simulink model "GLOSA_cs"  (Simulink 7.6)
settings: Solver=ode5  SolverMode=SingleTasking  StartTime=0.0  StopTime=40  FixedStep=1/100  RelTol=1e-3
PreLoadFcn:
  | check_NUTS_defined;
  | 
  | assignin('base',['model_closed_' bdroot],false);
InitFcn:
  | PreScanExperimentInitFcn(bdroot)
StopFcn:
  | PreScanExperimentStopFcn(bdroot)
undefined symbols (not defined in the file or in its folder): acceleration, actorID, actorType, DiscIntNeverNeededParam, GPS_alt, GPS_lat, GPS_long, intmax, lightBarInUse, messageID, publicVehicleType, rotZ, sireneInUse, trafficLightPriority, turnAdvice, velocity, x_ini_VRML, y_ini_VRML, yawRate, z_ini_VRML
libraries: simulink x337, simulink_extras x42, PreScanSimulinkLibrary x38, PreScanUsefulBlocks x14, simulink_need_slupdate x6
(part 3 of 38)

== system GLOSA_cs/Ford_Fiesta_1 ==
  SELF_Ford_Fiesta_1 : Inport  Tag=SELFInport
  Antenna_Rx_10 : Inport  Tag=AntennaRInport  Port=2
  Antenna_Rx_10_BusSelector : BusSelector  Tag=BusAntennaR  OutputSignals=warning,messageID,actorID,actorType,publicVehicleType,lightBarInUse,sireneInUse,velocity,acceleration,yawRate,turnAdvice,trafficLightPriority,GPS_lat,GPS_long,GPS_alt,rotZ
  Antenna_Rx_10_Demux : Subsystem  Tag=DemuxAntennaR  mask(PreScan Demux Block:){Channel=1}
      settings: DeleteFcn=PreScanBlockDeleteFcn;
      mask Display:
      | fprintf('Channel: %d',Channel)
      | port_label('output', 1, 'Vehicle_ID')
      | port_label('output', 2, 'GPS Pos Lat [deg]')
      | port_label('output', 3, 'GPS Pos Long [deg]')
      | port_label('output', 4, 'GPS Pos Alt [m]')
      | port_label('output', 5, 'Velocity [m/s]')
      | port_label('output', 6, 'Acceleration [m/s2]')
      | port_label('output', 7, 'Heading [deg]')
      | port_label('output', 8, 'Warning signal ID')
      | port_label('output', 9, 'Maneuvre ID')
      | port_label('output', 10, 'Vehicle Type')
      | port_label('output', 11, 'Braking Capacity')
      | port_label('output', 12, 'Vehicle Size')
      | port_label('output', 13, 'Spare')
  Antenna_Tx_10_BusAssignment : BusAssignment  Tag=BusAntennaT  AssignedSignals=warning,messageID,actorID,actorType,publicVehicleType,lightBarInUse,sireneInUse,velocity,acceleration,yawRate,turnAdvice,trafficLightPriority,GPS_lat,GPS_long,GPS_alt,rotZ
  Antenna_Tx_10_Mux : Subsystem  Tag=MuxAntennaT  mask(PreScan Mux Block:){Channel=1, TimeStep=1/100}
      mask prompts: TimeStep "Time Step [s]"
      settings: DeleteFcn=PreScanBlockDeleteFcn;
      mask Display:
      | fprintf('Channel: %d',Channel)
      | port_label('input', 1, 'Warning signal ID')
      | port_label('input', 2, 'Maneuvre ID')
      | port_label('input', 3, 'Vehicle Type')
      | port_label('input', 4, 'Braking Capacity')
      | port_label('input', 5, 'Vehicle Size')
      | port_label('input', 6, 'spare')
  Bus Selector : BusSelector  OutputSignals=Brake_[bar]
  Compare To Constant : simulink/Logic and Bit Operations/Compare To Constant  SystemSampleTime=-1  relop=>=  const=0.1  LogicOutDataTypeMode=uint8
  Constant1 : Constant  Value=15
  Constant2 : Constant  Value=15
  Data Type Conversion : DataTypeConversion
  Dynamics_Simple : Subsystem  Tag=Simple_Vehicle_Dynamics  mask(PreScan Block: Simple Dynamics){Migrate=off, TimeStep=1/100, ComputationMode=Continuous_Time}
      mask prompts: Migrate "Migrate. During migration this block will be replaced by a newer one."; TimeStep "Auto assigned: Time Step [s]"; ComputationMode "Computation mode:"
      mask help:
      | The Simple Dynamics Model is selected in the GUI to simulate vehicle dynamics behavior.
      |
      | The Simple Dynamics Model is completely open to the user. The parameters of this model can be set in the Simple Dynamics dialog in the GUI.
      |
      | For more information, please click Help.
      mask init:
      | p = SimpleDyn_pars;
      settings: DeleteFcn=PreScanBlockDeleteFcn;
      mask Help: eval('openHelp(220)')
      mask Display:
      | image(imread('SimpleDyn.png'))
      | port_label('input', 1, '{\delta}_S_t_ _e_e_r [deg]','texmode','on')
      | port_label('input', 2, 'Throttle [%]')
      | port_label('input', 3, 'Brake Pres [bar]')
      | port_label('input', 4, 'V_0 [ms-1]','texmode','on')
      | port_label('input', 5, 'Auto=[0]/Manual=[1]')
      | port_label('input', 6, 'Auto_GearShift')
      | port_label('input', 7, 'Manual_GearShift')
      | port_label('output', 1, 'State')
      | port_label('output', 2, 'Vx [ms-1]')
      | port_label('output', 3, 'Perf Params')
  GLOSA : Subsystem
  Gain : Gain  Gain=1/20
  Gain1 : Gain  Gain=1/20
  Ground_{1..22} (22 alike) : Ground
  Integer Delay{1..5} (5 alike) : simulink/Discrete/Integer Delay  NumDelays=1  InputProcessing=Inherited  vinit=0.0  samptime=-1
  Mux : Mux  Inputs=3
  PathFollower_1 : Subsystem  Tag=pathFollower  mask(PreScan Block: Path Follower){Migrate=on, FileName='Trajectory_4.mat', TunableSteeringGain=1, XShifting_wrt_COG=0}
      mask prompts: Migrate "Migrate. During migration this block will be replaced by a newer one."; FileName "Trajectory Name"; TunableSteeringGain "TunableSteeringGain [-]"; XShifting_wrt_COG "XShifting_wrt_COG [m]"
      mask help:
      | The Path Follower Model is selected in the GUI to simulate driver behavior.
      |
      | For more information, please click Help.
      mask init:
      | load(['trajectories/',eval(FileName)])
      | traject=trajectory';
      | 
      | Traj_x=traject(:,2);
      | Traj_y=traject(:,3);
      | Traj_z=traject(:,4);
      | Traj_vx=traject(:,8);
      | Traj_vy=traject(:,9);
      | Traj_vz=traject(:,10);
      mask Help: eval('openHelp(232)')
  Rate Limiter1 : RateLimiter  RisingSlewLimit=1.5  FallingSlewLimit=-1.5  SampleTimeMode=inherited  InitialCondition=15
  SELF_Demux : Subsystem  Tag=DemuxSELF
      settings: DeleteFcn=PreScanBlockDeleteFcn;
      mask Type: PreScan Demux Block:
      mask Display:
      | port_label('output',1,'x [m]')
      | port_label('output',2,'y [m]')
      | port_label('output',3,'z [m]','texmode','on')
      | port_label('output',4,'Rotx [deg]','texmode','on')
      | port_label('output',5,'Roty [deg]','texmode','on')
      | port_label('output',6,'Rotz [deg]','texmode','on')
      | port_label('output',7,'GPS lat [deg]','texmode','on')
      | port_label('output',8,'GPS long [deg]','texmode','on')
      | port_label('output',9,'GPS alt [m]','texmode','on')
      | port_label('output',10,'Velocity [m/s]')
      | port_label('output',11,'Heading [deg]')
      | port_label('output',12,'Yaw rate [deg/s]')
  Subsystem : Subsystem
  Terminator_{1..19} (19 alike) : Terminator
  TextureColor : Constant  Tag=TextureColor  Value="[0  0  0]"
  Trajectories : Subsystem  Tag=Trajectories
      settings: DeleteFcn=PreScanBlockDeleteFcn;
      mask Type: PreScan Source Block: Trajectories
      mask Description:
      | The Trajectory block contains the trajectories defined in the PreScan Experiment Editor. The output of the trajectory block is the (vehicle) State.
      | 
      | For more information, please click Help.
      mask Help: eval('openHelp(312)')
      mask Display: image(imread('Trajectory.png'));
  VISULightSource_Mux : Subsystem  Tag=MuxLightSource
      settings: DeleteFcn=PreScanBlockDeleteFcn;
      mask Type: PreScan Mux Block:
  VISUSteer_Mux : Subsystem  Tag=MuxSteer
      settings: DeleteFcn=PreScanBlockDeleteFcn;
      mask Type: PreScan Mux Block:
  VISUTextureColor_Mux : Subsystem  Tag=MuxSteer
      settings: DeleteFcn=PreScanBlockDeleteFcn;
      mask Type: PreScan Mux Block:
  VISUWheels_Mux : Subsystem  Tag=MuxWheels  mask(PreScan Mux Block:){TimeStep=1/100}
      mask prompts: TimeStep "Auto Assigned: Time Step [s]"
      settings: DeleteFcn=PreScanBlockDeleteFcn;
  pos : Constant  Tag=pos  Value=[271.5 5.25 180]
  vehicle CAM generator : Subsystem
  wheel Z disp : Subsystem  mask(Simple Dynamics parameters){a=1.17, b=1.77}
      mask prompts: a "Distance to the front wheel from cars center of gravity a [m]"; b "Distance to the back wheel from cars center of gravity b [m]"
  STATE_Ford_Fiesta_1 : Outport  Tag=StateOutport
  Antenna_Tx_10 : Outport  Tag=AntennaTOutport  Port=2
  VISULightSource_Ford_Fiesta_1 : Outport  Tag=VisuLightsOutport  Port=3  SampleTime=1/100
  VISUTextureColor_Ford_Fiesta_1 : Outport  Tag=VisuTextureColorOutport  Port=4  SampleTime=1/100
  VISUWheels_Ford_Fiesta_1 : Outport  Tag=VisuWheelsOutport  Port=5  SampleTime=1/100
  VISUSteer_Ford_Fiesta_1 : Outport  Tag=VisuSteerOutport  Port=6  SampleTime=1/100
  wires:
  Constant2/1 -> GLOSA/23(desiredVelocity)
  [Ford_Fiesta_1_GPS] -> vehicle CAM generator/10(GPS_Noise_src)
  [Ford_Fiesta_1_MUR] -> vehicle CAM generator/11(MessageUpdateRate)
  Bus Selector/1 -> Compare To Constant/1  "<Brake_[bar]>"
  Compare To Constant/1 -> Data Type Conversion/1
  [Ford_Fiesta_1_NOMM] -> GLOSA/24(NoMissedMessages)
  [Ford_Fiesta_1_TD] -> GLOSA/25(MessageDelay)
  GLOSA/1(velocity) -> Rate Limiter1/1
  Gain/1 -> Integer Delay3/1
  [Steer] -> Gain/1
  Gain1/1 -> Integer Delay2/1
  [Steer] -> Gain1/1
  [Vx_ms] -> Integer Delay5/1
  [Roty] -> wheel Z disp/1(rotY [deg])
  Rate Limiter1/1 -> Integer Delay4/1
  [Params] -> Bus Selector/1
  pos/1 -> PathFollower_1/1(TrajOffset_x,y,Y [m,m,deg])
  SELF_Ford_Fiesta_1/1 -> SELF_Demux/1(SELF)
  Antenna_Rx_10_Demux/13(spare) -> Antenna_Rx_10_BusSelector/1
  Antenna_Rx_10/1 -> Antenna_Rx_10_Demux/1(AntennaR)
  Antenna_Tx_10_Mux/2(Out2) -> [Antenna_Tx_10]
  [Antenna_Tx_10] -> Antenna_Tx_10_BusAssignment/1
  Antenna_Tx_10_Mux/1(Out1) -> Antenna_Tx_10/1
  VISULightSource_Mux/1(Lights) -> VISULightSource_Ford_Fiesta_1/1
  VISUTextureColor_Mux/1(TextureColor) -> VISUTextureColor_Ford_Fiesta_1/1
  TextureColor/1 -> VISUTextureColor_Mux/1(RGB [-,-,-])
  VISUWheels_Mux/1(Wheels) -> VISUWheels_Ford_Fiesta_1/1
  VISUSteer_Mux/1(Steer) -> VISUSteer_Ford_Fiesta_1/1
  PathFollower_1/2(throttle [%]) -> Dynamics_Simple/2(Throttle)
  PathFollower_1/3(brake pressure [bar]) -> Dynamics_Simple/3(Brake)
  PathFollower_1/4(v0 [m/s]) -> Dynamics_Simple/4(Initial_Vx_[ms-1])
  PathFollower_1/5(Auto_GearShift) -> Dynamics_Simple/6(Auto_GearShift)
  PathFollower_1/1(delta_steerwheel [deg]) -> Dynamics_Simple/1(DeltaSteer_[deg]), [Steer]
  vehicle CAM generator/16(CAM_rotZ) -> Antenna_Tx_10_BusAssignment/17
  vehicle CAM generator/15(CAM_GPSalt) -> Antenna_Tx_10_BusAssignment/16
  vehicle CAM generator/14(CAM_GPSlong) -> Antenna_Tx_10_BusAssignment/15
  vehicle CAM generator/13(CAM_GPSlat) -> Antenna_Tx_10_BusAssignment/14
  vehicle CAM generator/12(CAM_trafficLightPriority) -> Antenna_Tx_10_BusAssignment/13
  vehicle CAM generator/11(CAM_turnAdvice) -> Antenna_Tx_10_BusAssignment/12
  vehicle CAM generator/10(CAM_yawRate) -> Antenna_Tx_10_BusAssignment/11
  Antenna_Rx_10_BusSelector/16 -> GLOSA/22(CAM_rotZ_in)  "<rotZ>"
  Antenna_Rx_10_BusSelector/15 -> GLOSA/21(CAM_GPSalt_in)  "<GPS_alt>"
  Antenna_Rx_10_BusSelector/14 -> GLOSA/20(CAM_GPSlong_in)  "<GPS_long>"
  Antenna_Rx_10_BusSelector/13 -> GLOSA/19(CAM_GPSlat_in)  "<GPS_lat>"
  Antenna_Rx_10_BusSelector/12 -> GLOSA/18(CAM_trafficLightPriority_in)  "<trafficLightPriority>"
  Antenna_Rx_10_BusSelector/11 -> GLOSA/17(CAM_turnAdvice_in)  "<turnAdvice>"
  Antenna_Rx_10_BusSelector/10 -> GLOSA/16(CAM_yawRate_in)  "<yawRate>"
  Antenna_Rx_10_BusSelector/9 -> GLOSA/15(CAM_acceleration_in)  "<acceleration>"
  Antenna_Rx_10_BusSelector/8 -> GLOSA/14(CAM_velocity_in)  "<velocity>"
  Antenna_Rx_10_BusSelector/7 -> GLOSA/13(CAM_sireneInUse_in)  "<sireneInUse>"
  Antenna_Rx_10_BusSelector/6 -> GLOSA/12(CAM_lightBarInUse_in)  "<lightBarInUse>"
  Antenna_Rx_10_BusSelector/5 -> GLOSA/11(CAM_publicVehicleType_in)  "<publicVehicleType>"
  Antenna_Rx_10_BusSelector/4 -> GLOSA/10(CAM_vehicleType_in)  "<actorType>"
  Antenna_Rx_10_BusSelector/3 -> GLOSA/9(CAM_vehicleID_in)  "<actorID>"
  Antenna_Rx_10_BusSelector/2 -> GLOSA/8(CAM_messageID_in)  "<messageID>"
  Antenna_Rx_10_BusSelector/1 -> GLOSA/7(CAM_warning_in)  "<warning>"
  Constant1/1 -> PathFollower_1/4(Initial Velocity [m/s])
  Subsystem/1(Out1) -> STATE_Ford_Fiesta_1/1
  Mux/1 -> PathFollower_1/3(position x,y,Y [m,m,deg])
  SELF_Demux/12(yaw rate [deg/s]) -> vehicle CAM generator/9(yawRate), GLOSA/6(selfYawRate)
  SELF_Demux/10(velocity [m/s]) -> PathFollower_1/6(velocity [m/s]), vehicle CAM generator/8(velocity [m/s]), GLOSA/5(selfVelocity)
  SELF_Demux/9(GPS alt [m]) -> vehicle CAM generator/7(GPS_alt), GLOSA/3(selfGPSAltitude)
  SELF_Demux/8(GPS long [deg]) -> vehicle CAM generator/6(GPS_long), GLOSA/2(selfGPSLongitude)
  SELF_Demux/7(GPS lat [deg]) -> vehicle CAM generator/5(GPS_lat), GLOSA/1(selfGPSLatitude)
  SELF_Demux/6(rotz [deg]) -> Mux/3, vehicle CAM generator/4(rotZ [deg]), GLOSA/4(selfRotZ)
  SELF_Demux/3(z [m]) -> vehicle CAM generator/3(z [m])
  SELF_Demux/2(y [m]) -> Mux/2, vehicle CAM generator/2(y [m])
  SELF_Demux/1(x [m]) -> Mux/1, vehicle CAM generator/1(x [m])
  vehicle CAM generator/1(CAM_warning) -> Antenna_Tx_10_BusAssignment/2
  vehicle CAM generator/2(CAM_messageID) -> Antenna_Tx_10_BusAssignment/3
  vehicle CAM generator/3(CAM_vehicleID) -> Antenna_Tx_10_BusAssignment/4
  vehicle CAM generator/4(CAM_vehicleType) -> Antenna_Tx_10_BusAssignment/5
  vehicle CAM generator/5(CAM_publicVehicleType) -> Antenna_Tx_10_BusAssignment/6
  vehicle CAM generator/6(CAM_lightBarInUse) -> Antenna_Tx_10_BusAssignment/7
  vehicle CAM generator/7(CAM_sireneInUse) -> Antenna_Tx_10_BusAssignment/8
  vehicle CAM generator/8(CAM_velocity) -> Antenna_Tx_10_BusAssignment/9
  vehicle CAM generator/9(CAM_acceleration) -> Antenna_Tx_10_BusAssignment/10
  wheel Z disp/1(zDisp FL [m]) -> VISUWheels_Mux/4(zDisp FL [m])
  wheel Z disp/2(zDisp FR [m]) -> VISUWheels_Mux/5(zDisp FR [m])
  wheel Z disp/3(zDisp RL [m]) -> VISUWheels_Mux/6(zDisp RL [m])
  wheel Z disp/4(zDisp RR [m]) -> VISUWheels_Mux/7(zDisp RR [m])
  Data Type Conversion/1 -> VISULightSource_Mux/15(CombinedBrakeLightRight), VISULightSource_Mux/14(CombinedBrakeLightLeft), VISULightSource_Mux/11(BrakeLightMiddle)
  Integer Delay2/1 -> VISUWheels_Mux/1(Steer Angle Front [deg])
  Integer Delay5/1 -> VISUWheels_Mux/3(Velocity [m/s])
  Integer Delay3/1 -> VISUSteer_Mux/2(Steer [deg])
  SELF_Demux/5(roty [deg]) -> [Roty]
  Dynamics_Simple/3(Perf_Params) -> [Params]
  Integer Delay1/1 -> Antenna_Tx_10_Mux/6(spare)
  Integer Delay4/1 -> PathFollower_1/5(Desired Velocity [m/s])
  Dynamics_Simple/1(State) -> Subsystem/1(In1)
  Dynamics_Simple/2(Vx [m s-1]) -> [Vx_ms]
  Antenna_Tx_10_BusAssignment/1 -> Integer Delay1/1
  Antenna_Rx_10_Demux/1(Vehicle_ID) -> Terminator_1/1
  Antenna_Rx_10_Demux/2(GPS_pos_lat_[deg]) -> Terminator_2/1
  Antenna_Rx_10_Demux/3(GPS_pos_long_[deg]) -> Terminator_3/1
  Antenna_Rx_10_Demux/4(GPS_pos_alt_[m]) -> Terminator_4/1
  Antenna_Rx_10_Demux/5(Velocity_[m/s]) -> Terminator_5/1
  Antenna_Rx_10_Demux/6(Acceleration_[m/s2]) -> Terminator_6/1
  Antenna_Rx_10_Demux/7(Heading [deg]) -> Terminator_7/1
  Antenna_Rx_10_Demux/8(warning_signal_id) -> Terminator_8/1
  Antenna_Rx_10_Demux/9(maneuvre_id) -> Terminator_9/1
  Antenna_Rx_10_Demux/10(vehicle_type) -> Terminator_10/1
  Antenna_Rx_10_Demux/11(brakingcapacity) -> Terminator_11/1
  Antenna_Rx_10_Demux/12(vehicle_size) -> Terminator_12/1
  Ground_1/1 -> Antenna_Tx_10_Mux/1(warning_signal_id)
  Ground_2/1 -> Antenna_Tx_10_Mux/2(maneuvre_id)
  Ground_3/1 -> Antenna_Tx_10_Mux/3(vehicle_type)
  Ground_4/1 -> Antenna_Tx_10_Mux/4(brakingcapacity)
  Ground_5/1 -> Antenna_Tx_10_Mux/5(vehicle_size)
  Ground_6/1 -> Dynamics_Simple/5(AutoManual_OnOff)
  Ground_7/1 -> Dynamics_Simple/7(Manual_GearShift)
  GLOSA/2(Time) -> Terminator_13/1
  GLOSA/3(Light) -> Terminator_14/1
  Ground_8/1 -> PathFollower_1/2(ActorOffset_Left(+) / Right(-) [m])
  PathFollower_1/6(Actor/Traj_distance [m]) -> Terminator_15/1
  PathFollower_1/7(Actor/Traj_angle [deg]) -> Terminator_16/1
  SELF_Demux/4(rotx [deg]) -> Terminator_17/1
  SELF_Demux/11(heading [deg]) -> Terminator_18/1
  Trajectories/1(State) -> Terminator_19/1
  Ground_9/1 -> VISULightSource_Mux/1(IndicatorLightsLeft)
  Ground_10/1 -> VISULightSource_Mux/2(IndicatorLightsRight)
  Ground_11/1 -> VISULightSource_Mux/3(FogLightRearLeft)
  Ground_12/1 -> VISULightSource_Mux/4(FogLightRearRight)
  Ground_13/1 -> VISULightSource_Mux/5(FogLightFrontLeft)
  Ground_14/1 -> VISULightSource_Mux/6(FogLightFrontRight)
  Ground_15/1 -> VISULightSource_Mux/7(MainLightRearLeft)
  Ground_16/1 -> VISULightSource_Mux/8(MainLightRearRight)
  Ground_17/1 -> VISULightSource_Mux/9(MainLightFrontLeft)
  Ground_18/1 -> VISULightSource_Mux/10(MainLightFrontRight)
  Ground_19/1 -> VISULightSource_Mux/12(ReverseLightLeft)
  Ground_20/1 -> VISULightSource_Mux/13(ReverseLightRight)
  Ground_21/1 -> VISUSteer_Mux/1(Column [deg])
  Ground_22/1 -> VISUWheels_Mux/2(Steer Angle Rear [deg])

== system GLOSA_cs/GetSensors ==
  dummy : Inport
  GetSensors : M-S-Function  FunctionName=GetSensorsBlock  Parameters=TimeStep NrOfSensors
  tag scope: SimCoreSensors=global
  wires:
  GetSensors/1 -> [SimCoreSensors]
  dummy/1 -> GetSensors/1

== system GLOSA_cs/Mazda_RX8_1 ==
  SELF_Mazda_RX8_1 : Inport  Tag=SELFInport
  Antenna_Rx_8 : Inport  Tag=AntennaRInport  Port=2
  Antenna_Rx_8_BusSelector : BusSelector  Tag=BusAntennaR  OutputSignals=warning,messageID,actorID,actorType,publicVehicleType,lightBarInUse,sireneInUse,velocity,acceleration,yawRate,turnAdvice,trafficLightPriority,GPS_lat,GPS_long,GPS_alt,rotZ
  Antenna_Rx_8_Demux : Subsystem  Tag=DemuxAntennaR  mask(PreScan Demux Block:){Channel=1}
      settings: DeleteFcn=PreScanBlockDeleteFcn;
      mask Display:
      | fprintf('Channel: %d',Channel)
      | port_label('output', 1, 'Vehicle_ID')
      | port_label('output', 2, 'GPS Pos Lat [deg]')
      | port_label('output', 3, 'GPS Pos Long [deg]')
      | port_label('output', 4, 'GPS Pos Alt [m]')
      | port_label('output', 5, 'Velocity [m/s]')
      | port_label('output', 6, 'Acceleration [m/s2]')
      | port_label('output', 7, 'Heading [deg]')
      | port_label('output', 8, 'Warning signal ID')
      | port_label('output', 9, 'Maneuvre ID')
      | port_label('output', 10, 'Vehicle Type')
      | port_label('output', 11, 'Braking Capacity')
      | port_label('output', 12, 'Vehicle Size')
      | port_label('output', 13, 'Spare')
  Antenna_Tx_8_BusAssignment : BusAssignment  Tag=BusAntennaT  AssignedSignals=warning,messageID,actorID,actorType,publicVehicleType,lightBarInUse,sireneInUse,velocity,acceleration,yawRate,turnAdvice,trafficLightPriority,GPS_lat,GPS_long,GPS_alt,rotZ
  Antenna_Tx_8_Mux : Subsystem  Tag=MuxAntennaT  mask(PreScan Mux Block:){Channel=1, TimeStep=1/100}
      mask prompts: TimeStep "Time Step [s]"
      settings: DeleteFcn=PreScanBlockDeleteFcn;
      mask Display:
      | fprintf('Channel: %d',Channel)
      | port_label('input', 1, 'Warning signal ID')
      | port_label('input', 2, 'Maneuvre ID')
      | port_label('input', 3, 'Vehicle Type')
      | port_label('input', 4, 'Braking Capacity')
      | port_label('input', 5, 'Vehicle Size')
      | port_label('input', 6, 'spare')
  Bus Selector : BusSelector  OutputSignals=Brake_[bar]
  Compare To Constant : simulink/Logic and Bit Operations/Compare To Constant  SystemSampleTime=-1  relop=>=  const=0.1  LogicOutDataTypeMode=uint8
  Constant1 : Constant  Value=15
  Constant2 : Constant  Value=15
  Data Type Conversion : DataTypeConversion
  Dynamics_Simple : Subsystem  Tag=Simple_Vehicle_Dynamics  mask(PreScan Block: Simple Dynamics){Migrate=off, TimeStep=1/100, ComputationMode=Continuous_Time}
      mask prompts: Migrate "Migrate. During migration this block will be replaced by a newer one."; TimeStep "Auto assigned: Time Step [s]"; ComputationMode "Computation mode:"
      mask help:
      | The Simple Dynamics Model is selected in the GUI to simulate vehicle dynamics behavior.
      |
      | The Simple Dynamics Model is completely open to the user. The parameters of this model can be set in the Simple Dynamics dialog in the GUI.
      |
      | For more information, please click Help.
      mask init:
      | p = SimpleDyn_pars;
      settings: DeleteFcn=PreScanBlockDeleteFcn;
      mask Help: eval('openHelp(220)')
      mask Display:
      | image(imread('SimpleDyn.png'))
      | port_label('input', 1, '{\delta}_S_t_ _e_e_r [deg]','texmode','on')
      | port_label('input', 2, 'Throttle [%]')
      | port_label('input', 3, 'Brake Pres [bar]')
      | port_label('input', 4, 'V_0 [ms-1]','texmode','on')
      | port_label('input', 5, 'Auto=[0]/Manual=[1]')
      | port_label('input', 6, 'Auto_GearShift')
      | port_label('input', 7, 'Manual_GearShift')
      | port_label('output', 1, 'State')
      | port_label('output', 2, 'Vx [ms-1]')
      | port_label('output', 3, 'Perf Params')
  GLOSA : Subsystem
  Gain : Gain  Gain=1/20
  Gain1 : Gain  Gain=1/20
  Ground_{1..20} (20 alike) : Ground
  Integer Delay{1..5} (5 alike) : simulink/Discrete/Integer Delay  NumDelays=1  InputProcessing=Inherited  vinit=0.0  samptime=-1
  Mux : Mux  Inputs=3
  PathFollower_1 : Subsystem  Tag=pathFollower  mask(PreScan Block: Path Follower){Migrate=on, FileName='Trajectory_5.mat', TunableSteeringGain=1, XShifting_wrt_COG=0}
      mask prompts: Migrate "Migrate. During migration this block will be replaced by a newer one."; FileName "Trajectory Name"; TunableSteeringGain "TunableSteeringGain [-]"; XShifting_wrt_COG "XShifting_wrt_COG [m]"
      mask help:
      | The Path Follower Model is selected in the GUI to simulate driver behavior.
      |
      | For more information, please click Help.
      mask init:
      | load(['trajectories/',eval(FileName)])
      | traject=trajectory';
      | 
      | Traj_x=traject(:,2);
      | Traj_y=traject(:,3);
      | Traj_z=traject(:,4);
      | Traj_vx=traject(:,8);
      | Traj_vy=traject(:,9);
      | Traj_vz=traject(:,10);
      mask Help: eval('openHelp(232)')
  Rate Limiter1 : RateLimiter  RisingSlewLimit=1.5  FallingSlewLimit=-1.5  SampleTimeMode=inherited  InitialCondition=15
  Rate Transition2 : RateTransition  Integrity=off
  SELF_Demux : Subsystem  Tag=DemuxSELF
      settings: DeleteFcn=PreScanBlockDeleteFcn;
      mask Type: PreScan Demux Block:
      mask Display:
      | port_label('output',1,'x [m]')
      | port_label('output',2,'y [m]')
      | port_label('output',3,'z [m]','texmode','on')
      | port_label('output',4,'Rotx [deg]','texmode','on')
      | port_label('output',5,'Roty [deg]','texmode','on')
      | port_label('output',6,'Rotz [deg]','texmode','on')
      | port_label('output',7,'GPS lat [deg]','texmode','on')
      | port_label('output',8,'GPS long [deg]','texmode','on')
      | port_label('output',9,'GPS alt [m]','texmode','on')
      | port_label('output',10,'Velocity [m/s]')
      | port_label('output',11,'Heading [deg]')
      | port_label('output',12,'Yaw rate [deg/s]')
  Subsystem : Subsystem
  Terminator_{1..17} (17 alike) : Terminator
  TextureColor : Constant  Tag=TextureColor  Value="[255    0    0]"
  Trajectories : Subsystem  Tag=Trajectories
      settings: DeleteFcn=PreScanBlockDeleteFcn;
      mask Type: PreScan Source Block: Trajectories
      mask Description:
      | The Trajectory block contains the trajectories defined in the PreScan Experiment Editor. The output of the trajectory block is the (vehicle) State.
      | 
      | For more information, please click Help.
      mask Help: eval('openHelp(312)')
      mask Display: image(imread('Trajectory.png'));
  V2x_TC_GUI : Subsystem
  VISULightSource_Mux : Subsystem  Tag=MuxLightSource
      settings: DeleteFcn=PreScanBlockDeleteFcn;
      mask Type: PreScan Mux Block:
  VISUSteer_Mux : Subsystem  Tag=MuxSteer
      settings: DeleteFcn=PreScanBlockDeleteFcn;
      mask Type: PreScan Mux Block:
  VISUTextureColor_Mux : Subsystem  Tag=MuxSteer
      settings: DeleteFcn=PreScanBlockDeleteFcn;
      mask Type: PreScan Mux Block:
  VISUWheels_Mux : Subsystem  Tag=MuxWheels  mask(PreScan Mux Block:){TimeStep=1/100}
      mask prompts: TimeStep "Auto Assigned: Time Step [s]"
      settings: DeleteFcn=PreScanBlockDeleteFcn;
  pos : Constant  Tag=pos  Value=[-271.5 -5.2499999999999671 0]
  vehicle CAM generator : Subsystem
  wheel Z disp : Subsystem  mask(Simple Dynamics parameters){a=1.17, b=1.77}
      mask prompts: a "Distance to the front wheel from cars center of gravity a [m]"; b "Distance to the back wheel from cars center of gravity b [m]"
  STATE_Mazda_RX8_1 : Outport  Tag=StateOutport
  Antenna_Tx_8 : Outport  Tag=AntennaTOutport  Port=2
  VISULightSource_Mazda_RX8_1 : Outport  Tag=VisuLightsOutport  Port=3  SampleTime=1/100
  VISUTextureColor_Mazda_RX8_1 : Outport  Tag=VisuTextureColorOutport  Port=4  SampleTime=1/100
  VISUWheels_Mazda_RX8_1 : Outport  Tag=VisuWheelsOutport  Port=5  SampleTime=1/100
  VISUSteer_Mazda_RX8_1 : Outport  Tag=VisuSteerOutport  Port=6  SampleTime=1/100
  tag scope: perf_RX8=global
  wires:
  Constant2/1 -> GLOSA/23(desiredVelocity)
  [Mazda_RX8_1_GPS] -> vehicle CAM generator/10(GPS_Noise_src)
  [Mazda_RX8_1_MUR] -> vehicle CAM generator/11(MessageUpdateRate)
  [Mazda_RX8_1_NOMM] -> GLOSA/24(NoMissedMessages)
  [Mazda_RX8_1_TD] -> GLOSA/25(MessageDelay)
  Bus Selector/1 -> Compare To Constant/1  "<Brake_[bar]>"
  Compare To Constant/1 -> Data Type Conversion/1
  [perf_RX8] -> Rate Transition2/1
  Rate Transition2/1 -> V2x_TC_GUI/1(perf)
  GLOSA/2(Time) -> V2x_TC_GUI/3(lightsTime)
  GLOSA/3(Light) -> V2x_TC_GUI/2(lightsColor)
  GLOSA/1(velocity) -> Rate Limiter1/1
  Gain/1 -> Integer Delay3/1
  [Steer] -> Gain/1
  Rate Limiter1/1 -> Integer Delay1/1
  Integer Delay1/1 -> V2x_TC_GUI/4(suggestedVelocity), PathFollower_1/5(Desired Velocity [m/s])
  [perf_RX8] -> Bus Selector/1
  Gain1/1 -> Integer Delay2/1
  [Steer] -> Gain1/1
  [Vx_ms] -> Integer Delay5/1
  [Roty] -> wheel Z disp/1(rotY [deg])
  pos/1 -> PathFollower_1/1(TrajOffset_x,y,Y [m,m,deg])
  SELF_Mazda_RX8_1/1 -> SELF_Demux/1(SELF)
  Antenna_Rx_8_Demux/13(spare) -> Antenna_Rx_8_BusSelector/1
  Antenna_Rx_8/1 -> Antenna_Rx_8_Demux/1(AntennaR)
  Antenna_Tx_8_Mux/2(Out2) -> [Antenna_Tx_8]
  [Antenna_Tx_8] -> Antenna_Tx_8_BusAssignment/1
  Antenna_Tx_8_Mux/1(Out1) -> Antenna_Tx_8/1
  VISULightSource_Mux/1(Lights) -> VISULightSource_Mazda_RX8_1/1
  VISUTextureColor_Mux/1(TextureColor) -> VISUTextureColor_Mazda_RX8_1/1
  TextureColor/1 -> VISUTextureColor_Mux/1(RGB [-,-,-])
  VISUWheels_Mux/1(Wheels) -> VISUWheels_Mazda_RX8_1/1
  VISUSteer_Mux/1(Steer) -> VISUSteer_Mazda_RX8_1/1
  PathFollower_1/2(throttle [%]) -> Dynamics_Simple/2(Throttle)
  PathFollower_1/3(brake pressure [bar]) -> Dynamics_Simple/3(Brake)
  PathFollower_1/4(v0 [m/s]) -> Dynamics_Simple/4(Initial_Vx_[ms-1])
  PathFollower_1/5(Auto_GearShift) -> Dynamics_Simple/6(Auto_GearShift)
  PathFollower_1/1(delta_steerwheel [deg]) -> Dynamics_Simple/1(DeltaSteer_[deg]), [Steer]
  vehicle CAM generator/16(CAM_rotZ) -> Antenna_Tx_8_BusAssignment/17
  vehicle CAM generator/15(CAM_GPSalt) -> Antenna_Tx_8_BusAssignment/16
  vehicle CAM generator/14(CAM_GPSlong) -> Antenna_Tx_8_BusAssignment/15
  vehicle CAM generator/13(CAM_GPSlat) -> Antenna_Tx_8_BusAssignment/14
  vehicle CAM generator/12(CAM_trafficLightPriority) -> Antenna_Tx_8_BusAssignment/13
  vehicle CAM generator/11(CAM_turnAdvice) -> Antenna_Tx_8_BusAssignment/12
  vehicle CAM generator/9(CAM_acceleration) -> Antenna_Tx_8_BusAssignment/10
  Antenna_Rx_8_BusSelector/16 -> GLOSA/22(CAM_rotZ_in)  "<rotZ>"
  Antenna_Rx_8_BusSelector/15 -> GLOSA/21(CAM_GPSalt_in)  "<GPS_alt>"
  Antenna_Rx_8_BusSelector/14 -> GLOSA/20(CAM_GPSlong_in)  "<GPS_long>"
  Antenna_Rx_8_BusSelector/13 -> GLOSA/19(CAM_GPSlat_in)  "<GPS_lat>"
  Antenna_Rx_8_BusSelector/12 -> GLOSA/18(CAM_trafficLightPriority_in)  "<trafficLightPriority>"
  Antenna_Rx_8_BusSelector/11 -> GLOSA/17(CAM_turnAdvice_in)  "<turnAdvice>"
  Antenna_Rx_8_BusSelector/10 -> GLOSA/16(CAM_yawRate_in)  "<yawRate>"
  Antenna_Rx_8_BusSelector/9 -> GLOSA/15(CAM_acceleration_in)  "<acceleration>"
  Antenna_Rx_8_BusSelector/8 -> GLOSA/14(CAM_velocity_in)  "<velocity>"
  Antenna_Rx_8_BusSelector/7 -> GLOSA/13(CAM_sireneInUse_in)  "<sireneInUse>"
  Antenna_Rx_8_BusSelector/6 -> GLOSA/12(CAM_lightBarInUse_in)  "<lightBarInUse>"
  Antenna_Rx_8_BusSelector/5 -> GLOSA/11(CAM_publicVehicleType_in)  "<publicVehicleType>"
  Antenna_Rx_8_BusSelector/4 -> GLOSA/10(CAM_vehicleType_in)  "<actorType>"
  Antenna_Rx_8_BusSelector/3 -> GLOSA/9(CAM_vehicleID_in)  "<actorID>"
  Antenna_Rx_8_BusSelector/2 -> GLOSA/8(CAM_messageID_in)  "<messageID>"
  Antenna_Rx_8_BusSelector/1 -> GLOSA/7(CAM_warning_in)  "<warning>"
  Constant1/1 -> PathFollower_1/4(Initial Velocity [m/s])
  Subsystem/1(Out1) -> STATE_Mazda_RX8_1/1
  Mux/1 -> PathFollower_1/3(position x,y,Y [m,m,deg])
  SELF_Demux/12(yaw rate [deg/s]) -> vehicle CAM generator/9(yawRate), GLOSA/6(selfYawRate)
  SELF_Demux/10(velocity [m/s]) -> PathFollower_1/6(velocity [m/s]), vehicle CAM generator/8(velocity [m/s]), GLOSA/5(selfVelocity)
  SELF_Demux/9(GPS alt [m]) -> vehicle CAM generator/7(GPS_alt), GLOSA/3(selfGPSAltitude)
  SELF_Demux/8(GPS long [deg]) -> vehicle CAM generator/6(GPS_long), GLOSA/2(selfGPSLongitude)
  SELF_Demux/7(GPS lat [deg]) -> vehicle CAM generator/5(GPS_lat), GLOSA/1(selfGPSLatitude)
  SELF_Demux/6(rotz [deg]) -> Mux/3, vehicle CAM generator/4(rotZ [deg]), GLOSA/4(selfRotZ)
  SELF_Demux/3(z [m]) -> vehicle CAM generator/3(z [m])
  SELF_Demux/2(y [m]) -> Mux/2, vehicle CAM generator/2(y [m])
  SELF_Demux/1(x [m]) -> Mux/1, vehicle CAM generator/1(x [m])
  Dynamics_Simple/3(Perf_Params) -> [perf_RX8]
  vehicle CAM generator/1(CAM_warning) -> Antenna_Tx_8_BusAssignment/2
  vehicle CAM generator/2(CAM_messageID) -> Antenna_Tx_8_BusAssignment/3
  vehicle CAM generator/3(CAM_vehicleID) -> Antenna_Tx_8_BusAssignment/4
  vehicle CAM generator/4(CAM_vehicleType) -> Antenna_Tx_8_BusAssignment/5
  vehicle CAM generator/5(CAM_publicVehicleType) -> Antenna_Tx_8_BusAssignment/6
  vehicle CAM generator/6(CAM_lightBarInUse) -> Antenna_Tx_8_BusAssignment/7
  vehicle CAM generator/7(CAM_sireneInUse) -> Antenna_Tx_8_BusAssignment/8
  vehicle CAM generator/8(CAM_velocity) -> Antenna_Tx_8_BusAssignment/9
  vehicle CAM generator/10(CAM_yawRate) -> Antenna_Tx_8_BusAssignment/11
  wheel Z disp/1(zDisp FL [m]) -> VISUWheels_Mux/4(zDisp FL [m])
  wheel Z disp/2(zDisp FR [m]) -> VISUWheels_Mux/5(zDisp FR [m])
  wheel Z disp/3(zDisp RL [m]) -> VISUWheels_Mux/6(zDisp RL [m])
  wheel Z disp/4(zDisp RR [m]) -> VISUWheels_Mux/7(zDisp RR [m])
  Data Type Conversion/1 -> VISULightSource_Mux/13(BrakeLightRight), VISULightSource_Mux/12(BrakeLightMiddle), VISULightSource_Mux/11(BrakeLightLeft)
  Integer Delay3/1 -> VISUSteer_Mux/2(Steer [deg])
  Integer Delay5/1 -> VISUWheels_Mux/3(Velocity [m/s])
  Integer Delay2/1 -> VISUWheels_Mux/1(Steer Angle Front [deg])
  Integer Delay4/1 -> Antenna_Tx_8_Mux/6(spare)
  SELF_Demux/5(roty [deg]) -> [Roty]
  Dynamics_Simple/1(State) -> Subsystem/1(In1)
  Dynamics_Simple/2(Vx [m s-1]) -> [Vx_ms]
  Antenna_Tx_8_BusAssignment/1 -> Integer Delay4/1
  Antenna_Rx_8_Demux/1(Vehicle_ID) -> Terminator_1/1
  Antenna_Rx_8_Demux/2(GPS_pos_lat_[deg]) -> Terminator_2/1
  Antenna_Rx_8_Demux/3(GPS_pos_long_[deg]) -> Terminator_3/1
  Antenna_Rx_8_Demux/4(GPS_pos_alt_[m]) -> Terminator_4/1
  Antenna_Rx_8_Demux/5(Velocity_[m/s]) -> Terminator_5/1
  Antenna_Rx_8_Demux/6(Acceleration_[m/s2]) -> Terminator_6/1
  Antenna_Rx_8_Demux/7(Heading [deg]) -> Terminator_7/1
  Antenna_Rx_8_Demux/8(warning_signal_id) -> Terminator_8/1
  Antenna_Rx_8_Demux/9(maneuvre_id) -> Terminator_9/1
  Antenna_Rx_8_Demux/10(vehicle_type) -> Terminator_10/1
  Antenna_Rx_8_Demux/11(brakingcapacity) -> Terminator_11/1
  Antenna_Rx_8_Demux/12(vehicle_size) -> Terminator_12/1
  Ground_1/1 -> Antenna_Tx_8_Mux/1(warning_signal_id)
  Ground_2/1 -> Antenna_Tx_8_Mux/2(maneuvre_id)
  Ground_3/1 -> Antenna_Tx_8_Mux/3(vehicle_type)
  Ground_4/1 -> Antenna_Tx_8_Mux/4(brakingcapacity)
  Ground_5/1 -> Antenna_Tx_8_Mux/5(vehicle_size)
  Ground_6/1 -> Dynamics_Simple/5(AutoManual_OnOff)
  Ground_7/1 -> Dynamics_Simple/7(Manual_GearShift)
  Ground_8/1 -> PathFollower_1/2(ActorOffset_Left(+) / Right(-) [m])
  PathFollower_1/6(Actor/Traj_distance [m]) -> Terminator_13/1
  PathFollower_1/7(Actor/Traj_angle [deg]) -> Terminator_14/1
  SELF_Demux/4(rotx [deg]) -> Terminator_15/1
  SELF_Demux/11(heading [deg]) -> Terminator_16/1
  Trajectories/1(State) -> Terminator_17/1
  Ground_9/1 -> VISULightSource_Mux/1(IndicatorLightsLeft)
  Ground_10/1 -> VISULightSource_Mux/2(IndicatorLightsRight)
  Ground_11/1 -> VISULightSource_Mux/3(FogLightRearLeft)
  Ground_12/1 -> VISULightSource_Mux/4(FogLightRearRight)
  Ground_13/1 -> VISULightSource_Mux/5(FogLightFrontLeft)
  Ground_14/1 -> VISULightSource_Mux/6(FogLightFrontRight)
  Ground_15/1 -> VISULightSource_Mux/7(MainLightRearLeft)
  Ground_16/1 -> VISULightSource_Mux/8(MainLightRearRight)
  Ground_17/1 -> VISULightSource_Mux/9(MainLightFrontLeft)
  Ground_18/1 -> VISULightSource_Mux/10(MainLightFrontRight)
  Ground_19/1 -> VISUSteer_Mux/1(Column [deg])
  Ground_20/1 -> VISUWheels_Mux/2(Steer Angle Rear [deg])

== system GLOSA_cs/Noise Generator ==
  DISPLAY : M-S-Function  FunctionName=msfcn_GLOSA_PlotSpeed
  Memory{,1..23,30} (25 alike) : Memory
  tag scope: BMW_X5_1_GPS=global, BMW_X5_1_MUR=global, BMW_X5_1_NOMM=global, BMW_X5_1_TD=global, BMW_Z3_1_GPS=global, BMW_Z3_1_MUR=global, BMW_Z3_1_NOMM=global, BMW_Z3_1_TD=global, Ford_Fiesta_1_GPS=global, Ford_Fiesta_1_MUR=global, Ford_Fiesta_1_NOMM=global, Ford_Fiesta_1_TD=global, Mazda_RX8_1_GPS=global, Mazda_RX8_1_MUR=global, Mazda_RX8_1_NOMM=global, Mazda_RX8_1_TD=global, Toyota_Previa_1_GPS=global, Toyota_Previa_1_MUR=global, Toyota_Previa_1_NOMM=global, Toyota_Previa_1_TD=global, Toyota_Yaris_1_GPS=global, Toyota_Yaris_1_MUR=global, Toyota_Yaris_1_NOMM=global, Toyota_Yaris_1_TD=global
  wires:
  DISPLAY/4 -> [BMW_X5_1_TD], Memory18/1
  DISPLAY/3 -> [BMW_X5_1_NOMM], Memory19/1
  DISPLAY/2 -> [BMW_X5_1_MUR], Memory20/1
  DISPLAY/1 -> [BMW_X5_1_GPS], Memory21/1
  DISPLAY/5 -> [BMW_Z3_1_GPS], Memory17/1
  DISPLAY/6 -> [BMW_Z3_1_MUR], Memory16/1
  DISPLAY/7 -> [BMW_Z3_1_NOMM], Memory15/1
  DISPLAY/8 -> [BMW_Z3_1_TD], Memory14/1
  DISPLAY/9 -> [Toyota_Yaris_1_GPS], Memory13/1
  DISPLAY/10 -> [Toyota_Yaris_1_MUR], Memory12/1
  DISPLAY/11 -> [Toyota_Yaris_1_NOMM], Memory11/1
  DISPLAY/12 -> [Toyota_Yaris_1_TD], Memory10/1
  DISPLAY/13 -> [Mazda_RX8_1_GPS], Memory9/1
  DISPLAY/14 -> [Mazda_RX8_1_MUR], Memory8/1
  DISPLAY/15 -> [Mazda_RX8_1_NOMM], Memory7/1
  DISPLAY/16 -> [Mazda_RX8_1_TD], Memory6/1
  DISPLAY/17 -> [Toyota_Previa_1_GPS], Memory5/1
  DISPLAY/18 -> [Toyota_Previa_1_MUR], Memory4/1
  DISPLAY/19 -> [Toyota_Previa_1_NOMM], Memory3/1
  DISPLAY/20 -> [Toyota_Previa_1_TD], Memory2/1
  DISPLAY/21 -> [Ford_Fiesta_1_GPS], Memory1/1
  DISPLAY/22 -> [Ford_Fiesta_1_MUR], Memory23/1
  DISPLAY/23 -> [Ford_Fiesta_1_NOMM], Memory22/1
  DISPLAY/24 -> Memory/1, [Ford_Fiesta_1_TD]
  DISPLAY/25 -> Memory30/1
  Memory30/1 -> DISPLAY/25
  Memory/1 -> DISPLAY/24
  Memory1/1 -> DISPLAY/21
  Memory2/1 -> DISPLAY/20
  Memory3/1 -> DISPLAY/19
  Memory4/1 -> DISPLAY/18
  Memory5/1 -> DISPLAY/17
  Memory6/1 -> DISPLAY/16
  Memory7/1 -> DISPLAY/15
  Memory8/1 -> DISPLAY/14
  Memory9/1 -> DISPLAY/13
  Memory10/1 -> DISPLAY/12
  Memory11/1 -> DISPLAY/11
  Memory12/1 -> DISPLAY/10
  Memory13/1 -> DISPLAY/9
  Memory14/1 -> DISPLAY/8
  Memory15/1 -> DISPLAY/7
  Memory16/1 -> DISPLAY/6
  Memory17/1 -> DISPLAY/5
  Memory18/1 -> DISPLAY/4
  Memory19/1 -> DISPLAY/3
  Memory20/1 -> DISPLAY/2
  Memory21/1 -> DISPLAY/1
  Memory22/1 -> DISPLAY/23
  Memory23/1 -> DISPLAY/22

== system GLOSA_cs/RectangularBox_1 ==
  Antenna_Rx_2 : Inport  Tag=AntennaRInport
  Antenna_Rx_2_BusSelector : BusSelector  Tag=BusAntennaR  OutputSignals=warning,messageID,actorID,actorType,publicVehicleType,lightBarInUse,sireneInUse,velocity,acceleration,yawRate
  Antenna_Rx_2_Demux : Subsystem  Tag=DemuxAntennaR  mask(PreScan Demux Block:){Channel=1}
      settings: DeleteFcn=PreScanBlockDeleteFcn;
      mask Display:
      | fprintf('Channel: %d',Channel)
      | port_label('output', 1, 'Vehicle_ID')
      | port_label('output', 2, 'GPS Pos Lat [deg]')
      | port_label('output', 3, 'GPS Pos Long [deg]')
      | port_label('output', 4, 'GPS Pos Alt [m]')
      | port_label('output', 5, 'Velocity [m/s]')
      | port_label('output', 6, 'Acceleration [m/s2]')
      | port_label('output', 7, 'Heading [deg]')
      | port_label('output', 8, 'Warning signal ID')
      | port_label('output', 9, 'Maneuvre ID')
      | port_label('output', 10, 'Vehicle Type')
      | port_label('output', 11, 'Braking Capacity')
      | port_label('output', 12, 'Vehicle Size')
      | port_label('output', 13, 'Spare')
  Antenna_Tx_2_BusAssignment : BusAssignment  Tag=BusAntennaT  AssignedSignals=warning,messageID,actorID,actorType,publicVehicleType,lightBarInUse,sireneInUse,velocity,acceleration,yawRate
  Antenna_Tx_2_Mux : Subsystem  Tag=MuxAntennaT  mask(PreScan Mux Block:){Channel=1, TimeStep=1/100}
      mask prompts: TimeStep "Time Step [s]"
      settings: DeleteFcn=PreScanBlockDeleteFcn;
      mask Display:
      | fprintf('Channel: %d',Channel)
      | port_label('input', 1, 'Warning signal ID')
      | port_label('input', 2, 'Maneuvre ID')
      | port_label('input', 3, 'Vehicle Type')
      | port_label('input', 4, 'Braking Capacity')
      | port_label('input', 5, 'Vehicle Size')
      | port_label('input', 6, 'spare')
  Ground_{1..15} (15 alike) : Ground
  Integer Delay1 : simulink/Discrete/Integer Delay  NumDelays=1  InputProcessing=Inherited  vinit=0.0  samptime=-1
  Terminator_{1..22} (22 alike) : Terminator
  Antenna_Tx_2 : Outport  Tag=AntennaTOutport
  wires:
  Antenna_Rx_2_Demux/13(spare) -> Antenna_Rx_2_BusSelector/1
  Antenna_Rx_2/1 -> Antenna_Rx_2_Demux/1(AntennaR)
  Antenna_Tx_2_Mux/2(Out2) -> [Antenna_Tx_2]
  [Antenna_Tx_2] -> Antenna_Tx_2_BusAssignment/1
  Antenna_Tx_2_Mux/1(Out1) -> Antenna_Tx_2/1
  Antenna_Rx_2_BusSelector/1 -> Terminator_13/1  "<warning>"
  Antenna_Rx_2_BusSelector/2 -> Terminator_14/1  "<messageID>"
  Antenna_Rx_2_BusSelector/3 -> Terminator_15/1  "<actorID>"
  Antenna_Rx_2_BusSelector/4 -> Terminator_16/1  "<actorType>"
  Antenna_Rx_2_BusSelector/5 -> Terminator_17/1  "<publicVehicleType>"
  Antenna_Rx_2_BusSelector/6 -> Terminator_18/1  "<lightBarInUse>"
  Antenna_Rx_2_BusSelector/7 -> Terminator_19/1  "<sireneInUse>"
  Antenna_Rx_2_BusSelector/8 -> Terminator_20/1  "<velocity>"
  Antenna_Rx_2_BusSelector/9 -> Terminator_21/1  "<acceleration>"
  Antenna_Rx_2_BusSelector/10 -> Terminator_22/1  "<yawRate>"
  Integer Delay1/1 -> Antenna_Tx_2_Mux/6(spare)
  Antenna_Tx_2_BusAssignment/1 -> Integer Delay1/1
  Antenna_Rx_2_Demux/1(Vehicle_ID) -> Terminator_1/1
  Antenna_Rx_2_Demux/2(GPS_pos_lat_[deg]) -> Terminator_2/1
  Antenna_Rx_2_Demux/3(GPS_pos_long_[deg]) -> Terminator_3/1
  Antenna_Rx_2_Demux/4(GPS_pos_alt_[m]) -> Terminator_4/1
  Antenna_Rx_2_Demux/5(Velocity_[m/s]) -> Terminator_5/1
  Antenna_Rx_2_Demux/6(Acceleration_[m/s2]) -> Terminator_6/1
  Antenna_Rx_2_Demux/7(Heading [deg]) -> Terminator_7/1
  Antenna_Rx_2_Demux/8(warning_signal_id) -> Terminator_8/1
  Antenna_Rx_2_Demux/9(maneuvre_id) -> Terminator_9/1
  Antenna_Rx_2_Demux/10(vehicle_type) -> Terminator_10/1
  Antenna_Rx_2_Demux/11(brakingcapacity) -> Terminator_11/1
  Antenna_Rx_2_Demux/12(vehicle_size) -> Terminator_12/1
  Ground_1/1 -> Antenna_Tx_2_BusAssignment/2
  Ground_2/1 -> Antenna_Tx_2_BusAssignment/3
  Ground_3/1 -> Antenna_Tx_2_BusAssignment/4
  Ground_4/1 -> Antenna_Tx_2_BusAssignment/5
  Ground_5/1 -> Antenna_Tx_2_BusAssignment/6
  Ground_6/1 -> Antenna_Tx_2_BusAssignment/7
  Ground_7/1 -> Antenna_Tx_2_BusAssignment/8
  Ground_8/1 -> Antenna_Tx_2_BusAssignment/9
  Ground_9/1 -> Antenna_Tx_2_BusAssignment/10
  Ground_10/1 -> Antenna_Tx_2_BusAssignment/11
  Ground_11/1 -> Antenna_Tx_2_Mux/1(warning_signal_id)
  Ground_12/1 -> Antenna_Tx_2_Mux/2(maneuvre_id)
  Ground_13/1 -> Antenna_Tx_2_Mux/3(vehicle_type)
  Ground_14/1 -> Antenna_Tx_2_Mux/4(brakingcapacity)
  Ground_15/1 -> Antenna_Tx_2_Mux/5(vehicle_size)

== system GLOSA_cs/SELF_BMW_X5_1 ==
  Selector : Selector  IndexMode=Zero-based  IndexOptions=Starting index (dialog)  Indices=PortIndex  OutputSizes=PortWidth(1)
  U : Outport
  wires:
  Selector/1 -> U/1
  [SimCoreSensors] -> Selector/1

== system GLOSA_cs/SELF_BMW_Z3_1 ==
  Selector : Selector  IndexMode=Zero-based  IndexOptions=Starting index (dialog)  Indices=PortIndex  OutputSizes=PortWidth(1)
  U : Outport
  wires:
  Selector/1 -> U/1
  [SimCoreSensors] -> Selector/1

== system GLOSA_cs/SELF_Ford_Fiesta_1 ==
  Selector : Selector  IndexMode=Zero-based  IndexOptions=Starting index (dialog)  Indices=PortIndex  OutputSizes=PortWidth(1)
  U : Outport
  wires:
  Selector/1 -> U/1
  [SimCoreSensors] -> Selector/1

== system GLOSA_cs/SELF_Mazda_RX8_1 ==
  Selector : Selector  IndexMode=Zero-based  IndexOptions=Starting index (dialog)  Indices=PortIndex  OutputSizes=PortWidth(1)
  U : Outport
  wires:
  Selector/1 -> U/1
  [SimCoreSensors] -> Selector/1

== system GLOSA_cs/SELF_Sphere_1 ==
  Selector : Selector  IndexMode=Zero-based  IndexOptions=Starting index (dialog)  Indices=PortIndex  OutputSizes=PortWidth(1)
  U : Outport
  wires:
  Selector/1 -> U/1
  [SimCoreSensors] -> Selector/1

== system GLOSA_cs/SELF_Toyota_Previa_1 ==
  Selector : Selector  IndexMode=Zero-based  IndexOptions=Starting index (dialog)  Indices=PortIndex  OutputSizes=PortWidth(1)
  U : Outport
  wires:
  Selector/1 -> U/1
  [SimCoreSensors] -> Selector/1

== system GLOSA_cs/SELF_Toyota_Yaris_1 ==
  Selector : Selector  IndexMode=Zero-based  IndexOptions=Starting index (dialog)  Indices=PortIndex  OutputSizes=PortWidth(1)
  U : Outport
  wires:
  Selector/1 -> U/1
  [SimCoreSensors] -> Selector/1

== system GLOSA_cs/STATE_BMW_X5_1 ==
  State : Inport  SampleTime=TimeStep
  Acceleration : Subsystem  mask(ToSensorWorld){PortName=Acceleration_BMW_X5_1, PortIndex=6, PortWidth=3, TimeStep=1/100}
      mask prompts: PortName "Port Name [-]"; PortIndex "Index [-]"; PortWidth "Port Dimensions [-]"; TimeStep "Upload Period [s]"
      mask help: Sends signal(s) from Simulink to the Sensor World
      mask Help:
      | Sends signal(s) from Simulink to the Sensor World.
      | 
      | - Port Name [-]: specifies the name of the output terminal of the Simulink block.
      | - Index [-]: specifies the start position in the output vector sent to the Sensor World
      | - Port dimensions [-]: specifies the width (number of scalars) of the output port
      | - Upload period [s]: specifies the upload period (in seconds) of the port.
      mask Display: port_label('input', 1, PortName)
  Demux : Demux  Outputs=5
  Position : Subsystem  mask(ToSensorWorld){PortName=Position_BMW_X5_1, PortIndex=0, PortWidth=3, TimeStep=1/100}
      mask prompts: PortName "Port Name [-]"; PortIndex "Index [-]"; PortWidth "Port Dimensions [-]"; TimeStep "Upload Period [s]"
      mask help: Sends signal(s) from Simulink to the Sensor World
      mask Help:
      | Sends signal(s) from Simulink to the Sensor World.
      | 
      | - Port Name [-]: specifies the name of the output terminal of the Simulink block.
      | - Index [-]: specifies the start position in the output vector sent to the Sensor World
      | - Port dimensions [-]: specifies the width (number of scalars) of the output port
      | - Upload period [s]: specifies the upload period (in seconds) of the port.
      mask Display: port_label('input', 1, PortName)
  RPY : Subsystem  mask(ToSensorWorld){PortName=RPY_BMW_X5_1, PortIndex=9, PortWidth=3, TimeStep=1/100}
      mask prompts: PortName "Port Name [-]"; PortIndex "Index [-]"; PortWidth "Port Dimensions [-]"; TimeStep "Upload Period [s]"
      mask help: Sends signal(s) from Simulink to the Sensor World
      mask Help:
      | Sends signal(s) from Simulink to the Sensor World.
      | 
      | - Port Name [-]: specifies the name of the output terminal of the Simulink block.
      | - Index [-]: specifies the start position in the output vector sent to the Sensor World
      | - Port dimensions [-]: specifies the width (number of scalars) of the output port
      | - Upload period [s]: specifies the upload period (in seconds) of the port.
      mask Display: port_label('input', 1, PortName)
  Velocity : Subsystem  mask(ToSensorWorld){PortName=Velocity_BMW_X5_1, PortIndex=3, PortWidth=3, TimeStep=1/100}
      mask prompts: PortName "Port Name [-]"; PortIndex "Index [-]"; PortWidth "Port Dimensions [-]"; TimeStep "Upload Period [s]"
      mask help: Sends signal(s) from Simulink to the Sensor World
      mask Help:
      | Sends signal(s) from Simulink to the Sensor World.
      | 
      | - Port Name [-]: specifies the name of the output terminal of the Simulink block.
      | - Index [-]: specifies the start position in the output vector sent to the Sensor World
      | - Port dimensions [-]: specifies the width (number of scalars) of the output port
      | - Upload period [s]: specifies the upload period (in seconds) of the port.
      mask Display: port_label('input', 1, PortName)
  iniBODYAngles wrtVEH [rad] : Constant  Value=[Roll0 Pitch0 0]  SampleTime=TimeStep
  iniVEHAngles wrtGLOB [rad] : Constant  Value=[0 0 Yaw0]  SampleTime=TimeStep
  iniVEHposition wrtGLOB [m] : Constant  Value=[x0 y0 z0]  SampleTime=TimeStep
  state2FullState : PreScanSimulinkLibrary/state2FullState  SystemSampleTime=-1
  velRPY : Subsystem  mask(ToSensorWorld){PortName=velRPY_BMW_X5_1, PortIndex=12, PortWidth=3, TimeStep=1/100}
      mask prompts: PortName "Port Name [-]"; PortIndex "Index [-]"; PortWidth "Port Dimensions [-]"; TimeStep "Upload Period [s]"
      mask help: Sends signal(s) from Simulink to the Sensor World
      mask Help:
      | Sends signal(s) from Simulink to the Sensor World.
      | 
      | - Port Name [-]: specifies the name of the output terminal of the Simulink block.
      | - Index [-]: specifies the start position in the output vector sent to the Sensor World
      | - Port dimensions [-]: specifies the width (number of scalars) of the output port
      | - Upload period [s]: specifies the upload period (in seconds) of the port.
      mask Display: port_label('input', 1, PortName)
  wires:
  Demux/5 -> velRPY/1(Y)
  Demux/4 -> RPY/1(Y)
  Demux/3 -> Acceleration/1(Y)
  Demux/2 -> Velocity/1(Y)
  Demux/1 -> Position/1(Y)
  State/1 -> state2FullState/1
  state2FullState/1 -> Demux/1
  iniVEHposition wrtGLOB [m]/1 -> state2FullState/2
  iniBODYAngles wrtVEH [rad]/1 -> state2FullState/3
  iniVEHAngles wrtGLOB [rad]/1 -> state2FullState/4
